simulink model "HvacSnsrTFbFrnt"  (R2022b)
settings: Solver=FixedStepDiscrete  StartTime=0.0  StopTime=10.0  FixedStep=0.2  RelTol=1e-3
(part 2 of 2)

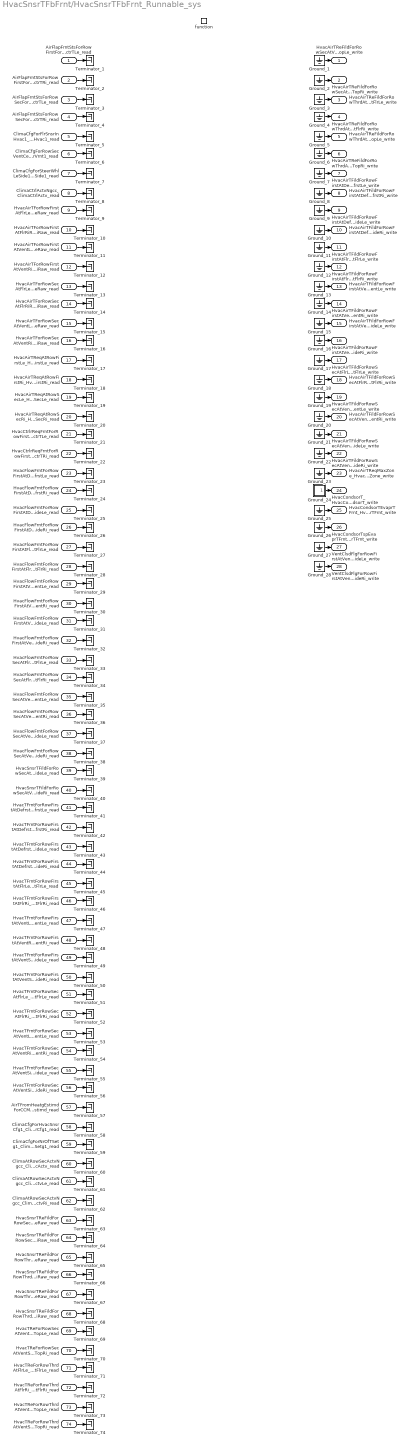
== system HvacSnsrTFbFrnt/HvacSnsrTFbFrnt_Runnable_sys ==
  AirFlapFrntStsForRowFirstForActrTLe_AirFlapFrntStsForRowFirstForActrTLe_read : Inport
  AirFlapFrntStsForRowFirstForActrTRi_AirFlapFrntStsForRowFirstForActrTRi_read : Inport  Port=2
  AirFlapFrntStsForRowSecForActrTLe_AirFlapFrntStsForRowSecForActrTLe_read : Inport  Port=3
  AirFlapFrntStsForRowSecForActrTRi_AirFlapFrntStsForRowSecForActrTRi_read : Inport  Port=4
  ClimaCfgForFlrSnsrInHvac1_ClimaCfgForFlrSnsrInHvac1_read : Inport  Port=5
  ClimaCfgForRowSecVentCentrVrnt1_ClimaCfgForRowSecVentCentrVrnt1_read : Inport  Port=6
  ClimaCfgForSteerWhlLeSide1_ClimaCfgForSteerWhlLeSide1_read : Inport  Port=7
  ClimaCtrlActvNgcc_ClimaCtrlActv_read : Inport  Port=8
  HvacAirTForRowFirstAtFlrLeRaw_HvacAirTForRowFirstAtFlrLeRaw_read : Inport  Port=9
  HvacAirTForRowFirstAtFlrRiRaw_HvacAirTForRowFirstAtFlrRiRaw_read : Inport  Port=10
  HvacAirTForRowFirstAtVentLeRaw_HvacAirTForRowFirstAtVentLeRaw_read : Inport  Port=11
  HvacAirTForRowFirstAtVentRiRaw_HvacAirTForRowFirstAtVentRiRaw_read : Inport  Port=12
  HvacAirTForRowSecAtFlrLeRaw_HvacAirTForRowSecAtFlrLeRaw_read : Inport  Port=13
  HvacAirTForRowSecAtFlrRiRaw_HvacAirTForRowSecAtFlrRiRaw_read : Inport  Port=14
  HvacAirTForRowSecAtVentLeRaw_HvacAirTForRowSecAtVentLeRaw_read : Inport  Port=15
  HvacAirTForRowSecAtVentRiRaw_HvacAirTForRowSecAtVentRiRaw_read : Inport  Port=16
  HvacAirTReqAtRowFirstLe_HvacAirTReqAtRowFirstLe_read : Inport  Port=17
  HvacAirTReqAtRowFirstRi_HvacAirTReqAtRowFirstRi_read : Inport  Port=18
  HvacAirTReqAtRowSecLe_HvacAirTReqAtRowSecLe_read : Inport  Port=19
  HvacAirTReqAtRowSecRi_HvacAirTReqAtRowSecRi_read : Inport  Port=20
  HvacCtrlrReqFrntForRowFirstForActrTLe_HvacCtrlrReqFrntForRowFirstForActrTLe_read : Inport  Port=21
  HvacCtrlrReqFrntForRowFirstForActrTRi_HvacCtrlrReqFrntForRowFirstForActrTRi_read : Inport  Port=22
  HvacFlowFrntForRowFirstAtDefrstLe_HvacFlowFrntForRowFirstAtDefrstLe_read : Inport  Port=23
  HvacFlowFrntForRowFirstAtDefrstRi_HvacFlowFrntForRowFirstAtDefrstRi_read : Inport  Port=24
  HvacFlowFrntForRowFirstAtDefrstSideLe_HvacFlowFrntForRowFirstAtDefrstSideLe_read : Inport  Port=25
  HvacFlowFrntForRowFirstAtDefrstSideRi_HvacFlowFrntForRowFirstAtDefrstSideRi_read : Inport  Port=26
  HvacFlowFrntForRowFirstAtFlrLe_HvacFlowFrntForRowFirstAtFlrLe_read : Inport  Port=27
  HvacFlowFrntForRowFirstAtFlrRi_HvacFlowFrntForRowFirstAtFlrRi_read : Inport  Port=28
  HvacFlowFrntForRowFirstAtVentLe_HvacFlowFrntForRowFirstAtVentLe_read : Inport  Port=29
  HvacFlowFrntForRowFirstAtVentRi_HvacFlowFrntForRowFirstAtVentRi_read : Inport  Port=30
  HvacFlowFrntForRowFirstAtVentSideLe_HvacFlowFrntForRowFirstAtVentSideLe_read : Inport  Port=31
  HvacFlowFrntForRowFirstAtVentSideRi_HvacFlowFrntForRowFirstAtVentSideRi_read : Inport  Port=32
  HvacFlowFrntForRowSecAtFlrLe_HvacFlowFrntForRowSecAtFlrLe_read : Inport  Port=33
  HvacFlowFrntForRowSecAtFlrRi_HvacFlowFrntForRowSecAtFlrRi_read : Inport  Port=34
  HvacFlowFrntForRowSecAtVentLe_HvacFlowFrntForRowSecAtVentLe_read : Inport  Port=35
  HvacFlowFrntForRowSecAtVentRi_HvacFlowFrntForRowSecAtVentRi_read : Inport  Port=36
  HvacFlowFrntForRowSecAtVentSideLe_HvacFlowFrntForRowSecAtVentSideLe_read : Inport  Port=37
  HvacFlowFrntForRowSecAtVentSideRi_HvacFlowFrntForRowSecAtVentSideRi_read : Inport  Port=38
  HvacSnsrTFildForRowSecAtVentSideLe_HvacSnsrTFildForRowSecAtVentSideLe_read : Inport  Port=39
  HvacSnsrTFildForRowSecAtVentSideRi_HvacSnsrTFildForRowSecAtVentSideRi_read : Inport  Port=40
  HvacTFrntForRowFirstAtDefrstLe_HvacTFrntForRowFirstAtDefrstLe_read : Inport  Port=41
  HvacTFrntForRowFirstAtDefrstRi_HvacTFrntForRowFirstAtDefrstRi_read : Inport  Port=42
  HvacTFrntForRowFirstAtDefrstSideLe_HvacTFrntForRowFirstAtDefrstSideLe_read : Inport  Port=43
  HvacTFrntForRowFirstAtDefrstSideRi_HvacTFrntForRowFirstAtDefrstSideRi_read : Inport  Port=44
  HvacTFrntForRowFirstAtFlrLe_HvacTFrntForRowFirstAtFlrLe_read : Inport  Port=45
  HvacTFrntForRowFirstAtFlrRi_HvacTFrntForRowFirstAtFlrRi_read : Inport  Port=46
  HvacTFrntForRowFirstAtVentLe_HvacTFrntForRowFirstAtVentLe_read : Inport  Port=47
  HvacTFrntForRowFirstAtVentRi_HvacTFrntForRowFirstAtVentRi_read : Inport  Port=48
  HvacTFrntForRowFirstAtVentSideLe_HvacTFrntForRowFirstAtVentSideLe_read : Inport  Port=49
  HvacTFrntForRowFirstAtVentSideRi_HvacTFrntForRowFirstAtVentSideRi_read : Inport  Port=50
  HvacTFrntForRowSecAtFlrLe_HvacTFrntForRowSecAtFlrLe_read : Inport  Port=51
  HvacTFrntForRowSecAtFlrRi_HvacTFrntForRowSecAtFlrRi_read : Inport  Port=52
  HvacTFrntForRowSecAtVentLe_HvacTFrntForRowSecAtVentLe_read : Inport  Port=53
  HvacTFrntForRowSecAtVentRi_HvacTFrntForRowSecAtVentRi_read : Inport  Port=54
  HvacTFrntForRowSecAtVentSideLe_HvacTFrntForRowSecAtVentSideLe_read : Inport  Port=55
  HvacTFrntForRowSecAtVentSideRi_HvacTFrntForRowSecAtVentSideRi_read : Inport  Port=56
  AirTFromHeatgEstimdForCCM_AirTFromHeatgEstimd_read : Inport  Port=57
  ClimaCfgForHvacSnsrCfg1_ClimaCfgForHvacSnsrCfg1_read : Inport  Port=58
  ClimaCfgForNrOfTSetg1_ClimaCfgForNrOfTSetg1_read : Inport  Port=59
  ClimaAtRowSecActvNgcc_ClimaAtRowSecActv_read : Inport  Port=60
  ClimaAtRowSecActvNgcc_ClimaAtRowSecActvLe_read : Inport  Port=61
  ClimaAtRowSecActvNgcc_ClimaAtRowSecActvRi_read : Inport  Port=62
  HvacSnsrTReFildForRowSecAtVentSideTopLeRaw_HvacSnsrTReFildForRowSecAtVentSideTopLeRaw_read : Inport  Port=63
  HvacSnsrTReFildForRowSecAtVentSideTopRiRaw_HvacSnsrTReFildForRowSecAtVentSideTopRiRaw_read : Inport  Port=64
  HvacSnsrTReFildForRowThrdAtFlrLeRaw_HvacSnsrTReFildForRowThrdAtFlrLeRaw_read : Inport  Port=65
  HvacSnsrTReFildForRowThrdAtFlrRiRaw_HvacSnsrTReFildForRowThrdAtFlrRiRaw_read : Inport  Port=66
  HvacSnsrTReFildForRowThrdAtVentSideTopLeRaw_HvacSnsrTReFildForRowThrdAtVentSideTopLeRaw_read : Inport  Port=67
  HvacSnsrTReFildForRowThrdAtVentSideTopRiRaw_HvacSnsrTReFildForRowThrdAtVentSideTopRiRaw_read : Inport  Port=68
  HvacTReForRowSecAtVentSideTopLe_HvacTReForRowSecAtVentSideTopLe_read : Inport  Port=69
  HvacTReForRowSecAtVentSideTopRi_HvacTReForRowSecAtVentSideTopRi_read : Inport  Port=70
  HvacTReForRowThrdAtFlrLe_HvacTReForRowThrdAtFlrLe_read : Inport  Port=71
  HvacTReForRowThrdAtFlrRi_HvacTReForRowThrdAtFlrRi_read : Inport  Port=72
  HvacTReForRowThrdAtVentSideTopLe_HvacTReForRowThrdAtVentSideTopLe_read : Inport  Port=73
  HvacTReForRowThrdAtVentSideTopRi_HvacTReForRowThrdAtVentSideTopRi_read : Inport  Port=74
  function : TriggerPort  TriggerType=function-call  VariantControl=(inherit)  SampleTimeType=periodic  SampleTime=-1
  Ground_{1..23,25..28} (27 alike) : Ground
  Ground_24 : Subsystem
  Terminator_{1..74} (74 alike) : Terminator
  HvacAirTReFildForRowSecAtVentSideTopLe_HvacAirTReFildForRowSecAtVentSideTopLe_write : Outport
      settings: EnsureOutportIsVirtual=on
  HvacAirTReFildForRowSecAtVentSideTopRi_HvacAirTReFildForRowSecAtVentSideTopRi_write : Outport  Port=2
      settings: EnsureOutportIsVirtual=on
  HvacAirTReFildForRowThrdAtFlrLe_HvacAirTReFildForRowThrdAtFlrLe_write : Outport  Port=3
      settings: EnsureOutportIsVirtual=on
  HvacAirTReFildForRowThrdAtFlrRi_HvacAirTReFildForRowThrdAtFlrRi_write : Outport  Port=4
      settings: EnsureOutportIsVirtual=on
  HvacAirTReFildForRowThrdAtVentSideTopLe_HvacAirTReFildForRowThrdAtVentSideTopLe_write : Outport  Port=5
      settings: EnsureOutportIsVirtual=on
  HvacAirTReFildForRowThrdAtVentSideTopRi_HvacAirTReFildForRowThrdAtVentSideTopRi_write : Outport  Port=6
      settings: EnsureOutportIsVirtual=on
  HvacAirTFildForRowFirstAtDefrstLe_HvacAirTFildForRowFirstAtDefrstLe_write : Outport  Port=7
      settings: EnsureOutportIsVirtual=on
  HvacAirTFildForRowFirstAtDefrstRi_HvacAirTFildForRowFirstAtDefrstRi_write : Outport  Port=8
      settings: EnsureOutportIsVirtual=on
  HvacAirTFildForRowFirstAtDefrstSideLe_HvacAirTFildForRowFirstAtDefrstSideLe_write : Outport  Port=9
      settings: EnsureOutportIsVirtual=on
  HvacAirTFildForRowFirstAtDefrstSideRi_HvacAirTFildForRowFirstAtDefrstSideRi_write : Outport  Port=10
      settings: EnsureOutportIsVirtual=on
  HvacAirTFildForRowFirstAtFlrLe_HvacAirTFildForRowFirstAtFlrLe_write : Outport  Port=11
      settings: EnsureOutportIsVirtual=on
  HvacAirTFildForRowFirstAtFlrRi_HvacAirTFildForRowFirstAtFlrRi_write : Outport  Port=12
      settings: EnsureOutportIsVirtual=on
  HvacAirTFildForRowFirstAtVentLe_HvacAirTFildForRowFirstAtVentLe_write : Outport  Port=13
      settings: EnsureOutportIsVirtual=on
  HvacAirTFildForRowFirstAtVentRi_HvacAirTFildForRowFirstAtVentRi_write : Outport  Port=14
      settings: EnsureOutportIsVirtual=on
  HvacAirTFildForRowFirstAtVentSideLe_HvacAirTFildForRowFirstAtVentSideLe_write : Outport  Port=15
      settings: EnsureOutportIsVirtual=on
  HvacAirTFildForRowFirstAtVentSideRi_HvacAirTFildForRowFirstAtVentSideRi_write : Outport  Port=16
      settings: EnsureOutportIsVirtual=on
  HvacAirTFildForRowSecAtFlrLe_HvacAirTFildForRowSecAtFlrLe_write : Outport  Port=17
      settings: EnsureOutportIsVirtual=on
  HvacAirTFildForRowSecAtFlrRi_HvacAirTFildForRowSecAtFlrRi_write : Outport  Port=18
      settings: EnsureOutportIsVirtual=on
  HvacAirTFildForRowSecAtVentLe_HvacAirTFildForRowSecAtVentLe_write : Outport  Port=19
      settings: EnsureOutportIsVirtual=on
  HvacAirTFildForRowSecAtVentRi_HvacAirTFildForRowSecAtVentRi_write : Outport  Port=20
      settings: EnsureOutportIsVirtual=on
  HvacAirTFildForRowSecAtVentSideLe_HvacAirTFildForRowSecAtVentSideLe_write : Outport  Port=21
      settings: EnsureOutportIsVirtual=on
  HvacAirTFildForRowSecAtVentSideRi_HvacAirTFildForRowSecAtVentSideRi_write : Outport  Port=22
      settings: EnsureOutportIsVirtual=on
  HvacAirTReqMaxZone_HvacAirTReqMaxZone_write : Outport  Port=23
      settings: EnsureOutportIsVirtual=on
  HvacCondsorT_HvacCondsorT_write : Outport  Port=24
      settings: EnsureOutportIsVirtual=on
  HvacCondsorTEvaprTFrnt_HvacCondsorTEvaprTFrnt_write : Outport  Port=25
      settings: EnsureOutportIsVirtual=on
  HvacCondsorTspEvaprTFrnt_HvacCondsorTspEvaprTFrnt_write : Outport  Port=26
      settings: EnsureOutportIsVirtual=on
  VentClsdFlgForRowFirstAtVentSideLe_VentClsdFlgForRowFirstAtVentSideLe_write : Outport  Port=27
      settings: EnsureOutportIsVirtual=on
  VentClsdFlgForRowFirstAtVentSideRi_VentClsdFlgForRowFirstAtVentSideRi_write : Outport  Port=28
      settings: EnsureOutportIsVirtual=on
  wires:
  AirFlapFrntStsForRowFirstForActrTLe_AirFlapFrntStsForRowFirstForActrTLe_read/1 -> Terminator_1/1
  AirFlapFrntStsForRowFirstForActrTRi_AirFlapFrntStsForRowFirstForActrTRi_read/1 -> Terminator_2/1
  AirFlapFrntStsForRowSecForActrTLe_AirFlapFrntStsForRowSecForActrTLe_read/1 -> Terminator_3/1
  AirFlapFrntStsForRowSecForActrTRi_AirFlapFrntStsForRowSecForActrTRi_read/1 -> Terminator_4/1
  ClimaCfgForFlrSnsrInHvac1_ClimaCfgForFlrSnsrInHvac1_read/1 -> Terminator_5/1
  ClimaCfgForRowSecVentCentrVrnt1_ClimaCfgForRowSecVentCentrVrnt1_read/1 -> Terminator_6/1
  ClimaCfgForSteerWhlLeSide1_ClimaCfgForSteerWhlLeSide1_read/1 -> Terminator_7/1
  ClimaCtrlActvNgcc_ClimaCtrlActv_read/1 -> Terminator_8/1
  HvacAirTForRowFirstAtFlrLeRaw_HvacAirTForRowFirstAtFlrLeRaw_read/1 -> Terminator_9/1
  HvacAirTForRowFirstAtFlrRiRaw_HvacAirTForRowFirstAtFlrRiRaw_read/1 -> Terminator_10/1
  HvacAirTForRowFirstAtVentLeRaw_HvacAirTForRowFirstAtVentLeRaw_read/1 -> Terminator_11/1
  HvacAirTForRowFirstAtVentRiRaw_HvacAirTForRowFirstAtVentRiRaw_read/1 -> Terminator_12/1
  HvacAirTForRowSecAtFlrLeRaw_HvacAirTForRowSecAtFlrLeRaw_read/1 -> Terminator_13/1
  HvacAirTForRowSecAtFlrRiRaw_HvacAirTForRowSecAtFlrRiRaw_read/1 -> Terminator_14/1
  HvacAirTForRowSecAtVentLeRaw_HvacAirTForRowSecAtVentLeRaw_read/1 -> Terminator_15/1
  HvacAirTForRowSecAtVentRiRaw_HvacAirTForRowSecAtVentRiRaw_read/1 -> Terminator_16/1
  HvacAirTReqAtRowFirstLe_HvacAirTReqAtRowFirstLe_read/1 -> Terminator_17/1
  HvacAirTReqAtRowFirstRi_HvacAirTReqAtRowFirstRi_read/1 -> Terminator_18/1
  HvacAirTReqAtRowSecLe_HvacAirTReqAtRowSecLe_read/1 -> Terminator_19/1
  HvacAirTReqAtRowSecRi_HvacAirTReqAtRowSecRi_read/1 -> Terminator_20/1
  HvacCtrlrReqFrntForRowFirstForActrTLe_HvacCtrlrReqFrntForRowFirstForActrTLe_read/1 -> Terminator_21/1
  HvacCtrlrReqFrntForRowFirstForActrTRi_HvacCtrlrReqFrntForRowFirstForActrTRi_read/1 -> Terminator_22/1
  HvacFlowFrntForRowFirstAtDefrstLe_HvacFlowFrntForRowFirstAtDefrstLe_read/1 -> Terminator_23/1
  HvacFlowFrntForRowFirstAtDefrstRi_HvacFlowFrntForRowFirstAtDefrstRi_read/1 -> Terminator_24/1
  HvacFlowFrntForRowFirstAtDefrstSideLe_HvacFlowFrntForRowFirstAtDefrstSideLe_read/1 -> Terminator_25/1
  HvacFlowFrntForRowFirstAtDefrstSideRi_HvacFlowFrntForRowFirstAtDefrstSideRi_read/1 -> Terminator_26/1
  HvacFlowFrntForRowFirstAtFlrLe_HvacFlowFrntForRowFirstAtFlrLe_read/1 -> Terminator_27/1
  HvacFlowFrntForRowFirstAtFlrRi_HvacFlowFrntForRowFirstAtFlrRi_read/1 -> Terminator_28/1
  HvacFlowFrntForRowFirstAtVentLe_HvacFlowFrntForRowFirstAtVentLe_read/1 -> Terminator_29/1
  HvacFlowFrntForRowFirstAtVentRi_HvacFlowFrntForRowFirstAtVentRi_read/1 -> Terminator_30/1
  HvacFlowFrntForRowFirstAtVentSideLe_HvacFlowFrntForRowFirstAtVentSideLe_read/1 -> Terminator_31/1
  HvacFlowFrntForRowFirstAtVentSideRi_HvacFlowFrntForRowFirstAtVentSideRi_read/1 -> Terminator_32/1
  HvacFlowFrntForRowSecAtFlrLe_HvacFlowFrntForRowSecAtFlrLe_read/1 -> Terminator_33/1
  HvacFlowFrntForRowSecAtFlrRi_HvacFlowFrntForRowSecAtFlrRi_read/1 -> Terminator_34/1
  HvacFlowFrntForRowSecAtVentLe_HvacFlowFrntForRowSecAtVentLe_read/1 -> Terminator_35/1
  HvacFlowFrntForRowSecAtVentRi_HvacFlowFrntForRowSecAtVentRi_read/1 -> Terminator_36/1
  HvacFlowFrntForRowSecAtVentSideLe_HvacFlowFrntForRowSecAtVentSideLe_read/1 -> Terminator_37/1
  HvacFlowFrntForRowSecAtVentSideRi_HvacFlowFrntForRowSecAtVentSideRi_read/1 -> Terminator_38/1
  HvacSnsrTFildForRowSecAtVentSideLe_HvacSnsrTFildForRowSecAtVentSideLe_read/1 -> Terminator_39/1
  HvacSnsrTFildForRowSecAtVentSideRi_HvacSnsrTFildForRowSecAtVentSideRi_read/1 -> Terminator_40/1
  HvacTFrntForRowFirstAtDefrstLe_HvacTFrntForRowFirstAtDefrstLe_read/1 -> Terminator_41/1
  HvacTFrntForRowFirstAtDefrstRi_HvacTFrntForRowFirstAtDefrstRi_read/1 -> Terminator_42/1
  HvacTFrntForRowFirstAtDefrstSideLe_HvacTFrntForRowFirstAtDefrstSideLe_read/1 -> Terminator_43/1
  HvacTFrntForRowFirstAtDefrstSideRi_HvacTFrntForRowFirstAtDefrstSideRi_read/1 -> Terminator_44/1
  HvacTFrntForRowFirstAtFlrLe_HvacTFrntForRowFirstAtFlrLe_read/1 -> Terminator_45/1
  HvacTFrntForRowFirstAtFlrRi_HvacTFrntForRowFirstAtFlrRi_read/1 -> Terminator_46/1
  HvacTFrntForRowFirstAtVentLe_HvacTFrntForRowFirstAtVentLe_read/1 -> Terminator_47/1
  HvacTFrntForRowFirstAtVentRi_HvacTFrntForRowFirstAtVentRi_read/1 -> Terminator_48/1
  HvacTFrntForRowFirstAtVentSideLe_HvacTFrntForRowFirstAtVentSideLe_read/1 -> Terminator_49/1
  HvacTFrntForRowFirstAtVentSideRi_HvacTFrntForRowFirstAtVentSideRi_read/1 -> Terminator_50/1
  HvacTFrntForRowSecAtFlrLe_HvacTFrntForRowSecAtFlrLe_read/1 -> Terminator_51/1
  HvacTFrntForRowSecAtFlrRi_HvacTFrntForRowSecAtFlrRi_read/1 -> Terminator_52/1
  HvacTFrntForRowSecAtVentLe_HvacTFrntForRowSecAtVentLe_read/1 -> Terminator_53/1
  HvacTFrntForRowSecAtVentRi_HvacTFrntForRowSecAtVentRi_read/1 -> Terminator_54/1
  HvacTFrntForRowSecAtVentSideLe_HvacTFrntForRowSecAtVentSideLe_read/1 -> Terminator_55/1
  HvacTFrntForRowSecAtVentSideRi_HvacTFrntForRowSecAtVentSideRi_read/1 -> Terminator_56/1
  AirTFromHeatgEstimdForCCM_AirTFromHeatgEstimd_read/1 -> Terminator_57/1
  ClimaCfgForHvacSnsrCfg1_ClimaCfgForHvacSnsrCfg1_read/1 -> Terminator_58/1
  ClimaCfgForNrOfTSetg1_ClimaCfgForNrOfTSetg1_read/1 -> Terminator_59/1
  ClimaAtRowSecActvNgcc_ClimaAtRowSecActv_read/1 -> Terminator_60/1
  ClimaAtRowSecActvNgcc_ClimaAtRowSecActvLe_read/1 -> Terminator_61/1
  ClimaAtRowSecActvNgcc_ClimaAtRowSecActvRi_read/1 -> Terminator_62/1
  HvacSnsrTReFildForRowSecAtVentSideTopLeRaw_HvacSnsrTReFildForRowSecAtVentSideTopLeRaw_read/1 -> Terminator_63/1
  HvacSnsrTReFildForRowSecAtVentSideTopRiRaw_HvacSnsrTReFildForRowSecAtVentSideTopRiRaw_read/1 -> Terminator_64/1
  HvacSnsrTReFildForRowThrdAtFlrLeRaw_HvacSnsrTReFildForRowThrdAtFlrLeRaw_read/1 -> Terminator_65/1
  HvacSnsrTReFildForRowThrdAtFlrRiRaw_HvacSnsrTReFildForRowThrdAtFlrRiRaw_read/1 -> Terminator_66/1
  HvacSnsrTReFildForRowThrdAtVentSideTopLeRaw_HvacSnsrTReFildForRowThrdAtVentSideTopLeRaw_read/1 -> Terminator_67/1
  HvacSnsrTReFildForRowThrdAtVentSideTopRiRaw_HvacSnsrTReFildForRowThrdAtVentSideTopRiRaw_read/1 -> Terminator_68/1
  HvacTReForRowSecAtVentSideTopLe_HvacTReForRowSecAtVentSideTopLe_read/1 -> Terminator_69/1
  HvacTReForRowSecAtVentSideTopRi_HvacTReForRowSecAtVentSideTopRi_read/1 -> Terminator_70/1
  HvacTReForRowThrdAtFlrLe_HvacTReForRowThrdAtFlrLe_read/1 -> Terminator_71/1
  HvacTReForRowThrdAtFlrRi_HvacTReForRowThrdAtFlrRi_read/1 -> Terminator_72/1
  HvacTReForRowThrdAtVentSideTopLe_HvacTReForRowThrdAtVentSideTopLe_read/1 -> Terminator_73/1
  HvacTReForRowThrdAtVentSideTopRi_HvacTReForRowThrdAtVentSideTopRi_read/1 -> Terminator_74/1
  Ground_1/1 -> HvacAirTReFildForRowSecAtVentSideTopLe_HvacAirTReFildForRowSecAtVentSideTopLe_write/1
  Ground_2/1 -> HvacAirTReFildForRowSecAtVentSideTopRi_HvacAirTReFildForRowSecAtVentSideTopRi_write/1
  Ground_3/1 -> HvacAirTReFildForRowThrdAtFlrLe_HvacAirTReFildForRowThrdAtFlrLe_write/1
  Ground_4/1 -> HvacAirTReFildForRowThrdAtFlrRi_HvacAirTReFildForRowThrdAtFlrRi_write/1
  Ground_5/1 -> HvacAirTReFildForRowThrdAtVentSideTopLe_HvacAirTReFildForRowThrdAtVentSideTopLe_write/1
  Ground_6/1 -> HvacAirTReFildForRowThrdAtVentSideTopRi_HvacAirTReFildForRowThrdAtVentSideTopRi_write/1
  Ground_7/1 -> HvacAirTFildForRowFirstAtDefrstLe_HvacAirTFildForRowFirstAtDefrstLe_write/1
  Ground_8/1 -> HvacAirTFildForRowFirstAtDefrstRi_HvacAirTFildForRowFirstAtDefrstRi_write/1
  Ground_9/1 -> HvacAirTFildForRowFirstAtDefrstSideLe_HvacAirTFildForRowFirstAtDefrstSideLe_write/1
  Ground_10/1 -> HvacAirTFildForRowFirstAtDefrstSideRi_HvacAirTFildForRowFirstAtDefrstSideRi_write/1
  Ground_11/1 -> HvacAirTFildForRowFirstAtFlrLe_HvacAirTFildForRowFirstAtFlrLe_write/1
  Ground_12/1 -> HvacAirTFildForRowFirstAtFlrRi_HvacAirTFildForRowFirstAtFlrRi_write/1
  Ground_13/1 -> HvacAirTFildForRowFirstAtVentLe_HvacAirTFildForRowFirstAtVentLe_write/1
  Ground_14/1 -> HvacAirTFildForRowFirstAtVentRi_HvacAirTFildForRowFirstAtVentRi_write/1
  Ground_15/1 -> HvacAirTFildForRowFirstAtVentSideLe_HvacAirTFildForRowFirstAtVentSideLe_write/1
  Ground_16/1 -> HvacAirTFildForRowFirstAtVentSideRi_HvacAirTFildForRowFirstAtVentSideRi_write/1
  Ground_17/1 -> HvacAirTFildForRowSecAtFlrLe_HvacAirTFildForRowSecAtFlrLe_write/1
  Ground_18/1 -> HvacAirTFildForRowSecAtFlrRi_HvacAirTFildForRowSecAtFlrRi_write/1
  Ground_19/1 -> HvacAirTFildForRowSecAtVentLe_HvacAirTFildForRowSecAtVentLe_write/1
  Ground_20/1 -> HvacAirTFildForRowSecAtVentRi_HvacAirTFildForRowSecAtVentRi_write/1
  Ground_21/1 -> HvacAirTFildForRowSecAtVentSideLe_HvacAirTFildForRowSecAtVentSideLe_write/1
  Ground_22/1 -> HvacAirTFildForRowSecAtVentSideRi_HvacAirTFildForRowSecAtVentSideRi_write/1
  Ground_23/1 -> HvacAirTReqMaxZone_HvacAirTReqMaxZone_write/1
  Ground_24/1(Out1) -> HvacCondsorT_HvacCondsorT_write/1
  Ground_25/1 -> HvacCondsorTEvaprTFrnt_HvacCondsorTEvaprTFrnt_write/1
  Ground_26/1 -> HvacCondsorTspEvaprTFrnt_HvacCondsorTspEvaprTFrnt_write/1
  Ground_27/1 -> VentClsdFlgForRowFirstAtVentSideLe_VentClsdFlgForRowFirstAtVentSideLe_write/1
  Ground_28/1 -> VentClsdFlgForRowFirstAtVentSideRi_VentClsdFlgForRowFirstAtVentSideRi_write/1

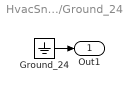
== system HvacSnsrTFbFrnt/HvacSnsrTFbFrnt_Runnable_sys/Ground_24 ==
  Ground_24 : Ground
  Out1 : Outport  OutDataTypeStr=Bus: EvaprTFrnt  PortDimensions=1
  wires:
  Ground_24/1 -> Out1/1
